simulink model "v13_na_ri_8r128dr_ver101"  (Simulink 7.6)
settings: Solver=ode45  StartTime=0.0  StopTime=10000  RelTol=1e-3
libraries: xbsIndex_r4 x1533, xps_library x40, casper_library_misc x20, dspsigops x16, simulink x7, casper_library_flow_control x4
(part 1 of 15)
settings Model: Creator=dave  LastModifiedBy=chenhong
settings OptimizationCC: InitFltsAndDblsToZero=on
settings DebuggingCC: StrictBusMsg=Warning

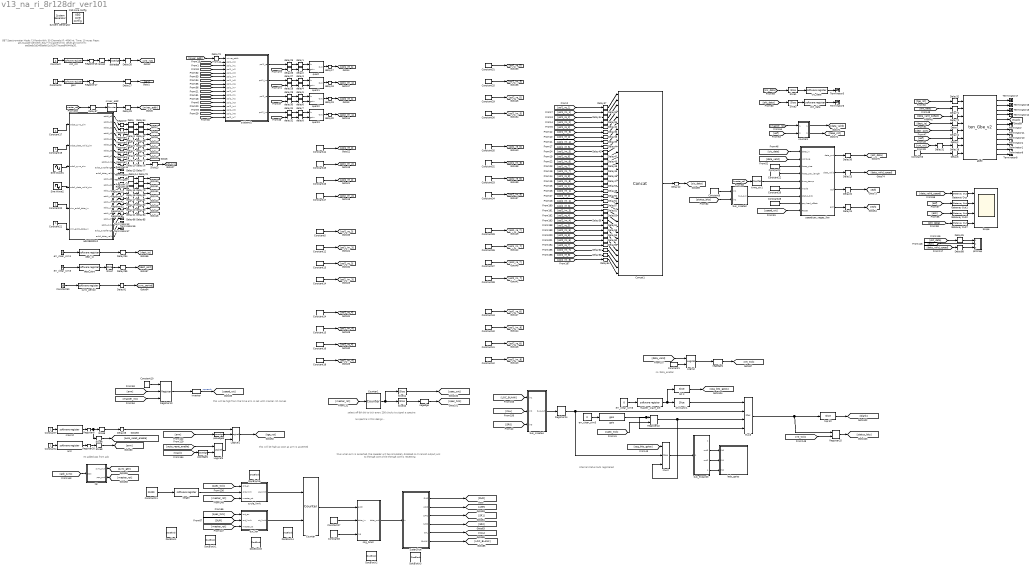
== system v13_na_ri_8r128dr_ver101 ==
  note: GBT Spectrometer
      | Mode: 13
      | Bandwidth: 30
      | Channels/IF: 4096
      | Int. Time: 10 msec
      | Repo: git://casper.berkeley.edu/~mwagner/mlib_devel.git
      | Commit: ae0be6d1d34f5befa01c913b77ecae840440e2f1
  note: internal status buts registered
  note: r6: added pps from adc
  note: this will be high from the time arm is set until master rst comes
  note: this will be high as soon as arm is asserted
  note: thus when arm is asserted, the speader will be completely disabled so it cannot output junk to the tge core while the tge core is resetting
  note: select off 8th bit to tick every 256 clocks to signal a spectra
  note: no 'data_enable'
  note: no spectra in this design...
  " System Generator" : xbsIndex_r4/ System Generator  Tag=genX  SystemSampleTime=-1  xilinxfamily=virtex5  part=xc5vsx95t  speed=-1  package=ff1136  synthesis_tool=XST  clock_wrapper=Clock Enables  directory=./mode13_1sub_no_cic/sysgen  proj_type=Project Navigator  Synth_file=XST Defaults  Impl_file=ISE Defaults  testbench=off  simulink_period=1  sysclk_period=6.6667  dcm_input_clock_period=100  incr_netlist=off  trim_vbits=Everywhere in SubSystem  dbl_ovrd=According to Block Masks  core_generation=According to Block Masks  run_coregen=off  deprecated_control=off  eval_field=0  block_type=sysgen
  Concat1 : xbsIndex_r4/Concat  num_inputs=32  dbl_ovrd=off  block_type=concat
  Constant{,3..4,7,17,19..21} (8 alike) : Constant
  Constant1 : xbsIndex_r4/Constant  const=1  gui_display_data_type=Boolean  arith_type=Boolean  n_bits=8  bin_pt=0  preci_type=Single  exp_width=8  frac_width=24  explicit_period=on  period=1  equ=P=C  opselect=C  inp2=PCIN>>17  opr=+  inp1=P  carry=CIN  dbl_ovrd=off  block_type=constant
  Constant10 : xbsIndex_r4/Constant  const=1  gui_display_data_type=Fixed-point  arith_type=Boolean  n_bits=16  bin_pt=0  preci_type=Single  exp_width=8  frac_width=24  explicit_period=on  period=1  equ=P=C  opselect=C  inp2=PCIN>>17  opr=+  inp1=P  carry=CIN  dbl_ovrd=off  block_type=constant
      settings: block_version=11.4
  Constant11 : Constant  Value=21200  VectorParams1D=off
  Constant{5,12..16,18,24..25,29..47} (28 alike) : xbsIndex_r4/Constant  const=0  gui_display_data_type=Fixed-point  arith_type=Unsigned  n_bits=8  bin_pt=0  preci_type=Single  exp_width=8  frac_width=24  explicit_period=on  period=1  equ=P=C  opselect=C  inp2=PCIN>>17  opr=+  inp1=P  carry=CIN  dbl_ovrd=off  block_type=constant
  Constant2 : xbsIndex_r4/Constant  const=8192+4*8  gui_display_data_type=Fixed-point  arith_type=Unsigned  n_bits=40  bin_pt=0  preci_type=Single  exp_width=8  frac_width=24  explicit_period=on  period=1  equ=P=C  opselect=C  inp2=PCIN>>17  opr=+  inp1=P  carry=CIN  dbl_ovrd=off  block_type=constant
  Constant22 : xbsIndex_r4/Constant  const=8192  gui_display_data_type=Fixed-point  arith_type=Unsigned  n_bits=40  bin_pt=0  preci_type=Single  exp_width=8  frac_width=24  explicit_period=on  period=1  equ=P=C  opselect=C  inp2=PCIN>>17  opr=+  inp1=P  carry=CIN  dbl_ovrd=off  block_type=constant
  Constant26 : xbsIndex_r4/Constant  const=13  gui_display_data_type=Fixed-point  arith_type=Unsigned  n_bits=40  bin_pt=0  preci_type=Single  exp_width=8  frac_width=24  explicit_period=on  period=1  equ=P=C  opselect=C  inp2=PCIN>>17  opr=+  inp1=P  carry=CIN  dbl_ovrd=off  block_type=constant
  Constant27 : xbsIndex_r4/Constant  const=0  gui_display_data_type=Fixed-point  arith_type=Unsigned  n_bits=32  bin_pt=0  preci_type=Single  exp_width=8  frac_width=24  explicit_period=on  period=1  equ=P=C  opselect=C  inp2=PCIN>>17  opr=+  inp1=P  carry=CIN  dbl_ovrd=off  block_type=constant
      settings: block_version=11.3
  Constant28 : xbsIndex_r4/Constant  const=0  gui_display_data_type=Fixed-point  arith_type=Unsigned  n_bits=40  bin_pt=0  preci_type=Single  exp_width=8  frac_width=24  explicit_period=on  period=1  equ=P=C  opselect=C  inp2=PCIN>>17  opr=+  inp1=P  carry=CIN  dbl_ovrd=off  block_type=constant
  Constant50 : Constant  Value=16
  Constant6 : xbsIndex_r4/Constant  const=0  gui_display_data_type=Fixed-point  arith_type=Boolean  n_bits=1  bin_pt=0  preci_type=Single  exp_width=8  frac_width=24  explicit_period=on  period=1  equ=P=C  opselect=C  inp2=PCIN>>17  opr=+  inp1=P  carry=CIN  dbl_ovrd=off  block_type=constant
  Constant8 : xbsIndex_r4/Constant  const=0  gui_display_data_type=Fixed-point  arith_type=Boolean  n_bits=32  bin_pt=0  preci_type=Single  exp_width=8  frac_width=24  explicit_period=on  period=1  equ=P=C  opselect=C  inp2=PCIN>>17  opr=+  inp1=P  carry=CIN  dbl_ovrd=off  block_type=constant
      settings: block_version=11.3
  Constant9 : xbsIndex_r4/Constant  const=0  gui_display_data_type=Fixed-point  arith_type=Unsigned  n_bits=36  bin_pt=0  preci_type=Single  exp_width=8  frac_width=24  explicit_period=on  period=1  equ=P=C  opselect=C  inp2=PCIN>>17  opr=+  inp1=P  carry=CIN  dbl_ovrd=off  block_type=constant
  Counter : xbsIndex_r4/Counter  cnt_type=Free Running  cnt_to=Inf  operation=Up  start_count=0  cnt_by_val=1  arith_type=Unsigned  n_bits=8  bin_pt=0  load_pin=off  rst=on  en=on  explicit_period=on  period=1  dbl_ovrd=off  use_behavioral_HDL=off  implementation=DSP48  block_type=counter
      settings: block_version=11.3
  Counter1 : xbsIndex_r4/Counter  cnt_type=Free Running  cnt_to=Inf  operation=Up  start_count=0  cnt_by_val=1  arith_type=Unsigned  n_bits=48  bin_pt=0  load_pin=off  rst=on  en=off  explicit_period=on  period=1  dbl_ovrd=off  use_behavioral_HDL=off  implementation=DSP48  block_type=counter
      settings: block_version=11.3
  Delay{,3..5,7..10,13..17,19..24,30..31,66} (22 alike) : xbsIndex_r4/Delay  rst=off  infoeditControl=Selection of Reset will increase slice count due to use of real FFs and instead of SRLs  en=off  latency=1  dbl_ovrd=off  reg_retiming=on  block_type=delay
      Delay settings: block_version=11.4
      Delay10 settings: block_version=11.4
      Delay13 settings: block_version=11.4
      Delay14 settings: block_version=11.4
      Delay15 settings: block_version=11.4
      Delay16 settings: block_version=11.4
      Delay17 settings: block_version=11.4
      Delay19 settings: block_version=11.4
      Delay20 settings: block_version=11.4
      Delay21 settings: block_version=11.4
      Delay3 settings: block_version=11.4
      Delay4 settings: block_version=11.4
      Delay5 settings: block_version=11.4
      Delay66 settings: block_version=11.4
      Delay7 settings: block_version=11.4
      Delay8 settings: block_version=11.4
      Delay9 settings: block_version=11.4
  Delay{1..2,6,11,18,28,32,71..72} (9 alike) : xbsIndex_r4/Delay  rst=off  infoeditControl=Selection of Reset will increase slice count due to use of real FFs and instead of SRLs  en=off  latency=8  dbl_ovrd=off  reg_retiming=on  block_type=delay
  Delay{12,33..53,55..65} (33 alike) : xbsIndex_r4/Delay  rst=off  infoeditControl=Selection of Reset will increase slice count due to use of real FFs and instead of SRLs  en=off  latency=4  dbl_ovrd=off  reg_retiming=on  block_type=delay
      settings: block_version=11.4
  Delay25 : xbsIndex_r4/Delay  rst=off  infoeditControl=Selection of Reset will increase slice count due to use of real FFs and instead of SRLs  en=off  latency=12  dbl_ovrd=off  reg_retiming=on  block_type=delay
  Delay{26..27,29,73} (4 alike) : xbsIndex_r4/Delay  rst=off  infoeditControl=Selection of Reset will increase slice count due to use of real FFs and instead of SRLs  en=off  latency=2  dbl_ovrd=off  reg_retiming=on  block_type=delay
      settings: block_version=11.4
  Delay{87,91,294..295} (4 alike) : xbsIndex_r4/Delay  rst=off  infoeditControl=Selection of Reset will increase slice count due to use of real FFs and instead of SRLs  en=off  latency=1  dbl_ovrd=off  reg_retiming=off  block_type=delay
      settings: block_version=11.4
  Delay{54,67..70,74..85} (17 alike) : xbsIndex_r4/Delay  rst=off  infoeditControl=Selection of Reset will increase slice count due to use of real FFs and instead of SRLs  en=off  latency=3  dbl_ovrd=off  reg_retiming=on  block_type=delay
      settings: block_version=11.4
  Delay88 : xbsIndex_r4/Delay  rst=off  infoeditControl=Selection of Reset will increase slice count due to use of real FFs and instead of SRLs  en=off  latency=12  dbl_ovrd=off  reg_retiming=on  block_type=delay
  DocBlock{,1..4,6,8} (7 alike) : simulink/Model-Wide Utilities/DocBlock  SystemSampleTime=-1  DocumentType=Text
  Gateway Out{,1..2,7} (4 alike) : xbsIndex_r4/Gateway Out  hdl_port=on  timing_constraint=None  locs_specified=off  LOCs={}  block_type=gatewayout
      settings: infoedit="Gateway out block.  Converts Xilinx fixed point inputs into ouputs of type Simulink integer, double, or fixed point.<br><br>Hardware notes:  In hardware these blocks become top level output ports or are discarded, depending on how they are configured."  block_version=11.4
  Inverter : xbsIndex_r4/Inverter  en=off  latency=0  dbl_ovrd=off  block_type=inv
      settings: block_version=11.4
  Logical : xbsIndex_r4/Logical  logical_function=AND  inputs=2  en=off  latency=0  precision=Full  arith_type=Unsigned  n_bits=16  bin_pt=0  align_bp=on  dbl_ovrd=off  block_type=logical
      settings: block_version=11.4
  Logical2 : xbsIndex_r4/Logical  logical_function=OR  inputs=3  en=off  latency=0  precision=Full  arith_type=Unsigned  n_bits=8  bin_pt=2  align_bp=on  dbl_ovrd=off  block_type=logical
      settings: xl_area=[0, 0, 0, 0, 0, 0, 0]
  Logical3 : xbsIndex_r4/Logical  logical_function=AND  inputs=2  en=off  latency=1  precision=Full  arith_type=Unsigned  n_bits=8  bin_pt=2  align_bp=on  dbl_ovrd=off  block_type=logical
      settings: xl_area=[0, 0, 0, 0, 0, 0, 0]
  Mux2 : xbsIndex_r4/Mux  inputs=2  en=off  latency=0  precision=Full  arith_type=Unsigned  n_bits=16  bin_pt=14  quantization=Truncate  overflow=Wrap  dbl_ovrd=off  block_type=mux
  Mux3 : xbsIndex_r4/Mux  inputs=3  en=off  latency=0  precision=Full  arith_type=Unsigned  n_bits=16  bin_pt=14  quantization=Truncate  overflow=Wrap  dbl_ovrd=off  block_type=mux
  Register{,1..8,12..13,16..17,20,24,42..44,46..47,52,61} (22 alike) : xbsIndex_r4/Register  init=0  rst=off  en=off  dbl_ovrd=off  block_type=register
      settings: block_version=11.4
  Register10 : xbsIndex_r4/Register  init=0  rst=on  en=on  dbl_ovrd=off  block_type=register
      settings: block_version=11.4
  Register22 : xbsIndex_r4/Register  init=0  rst=off  en=on  dbl_ovrd=off  block_type=register
      settings: block_version=11.4
  Register25 : xbsIndex_r4/Register  init=0  rst=off  en=on  dbl_ovrd=off  block_type=register
      settings: block_version=11.4
  Scope : Scope  NumInputPorts=4
      settings: TimeRange=6000  YMin=0~-1~0~0  YMax=1~1~300000000000000~1e+19  MaxDataPoints=1000000
  Sine Wave1 : Sin  Amplitude=.9  Frequency=2.*pi/4.  SampleTime=.0625
  Sine Wave2 : Sin  Amplitude=.9  Frequency=pi/2  Phase=2*pi*240/200  SampleTime=.0625
  Slice1 : xbsIndex_r4/Slice  nbits=32  boolean_output=off  mode=Lower Bit Location + Width  bit1=0  base1=MSB of Input  bit0=0  base0=LSB of Input  dbl_ovrd=off  block_type=slice
  Slice10 : xbsIndex_r4/Slice  nbits=1  boolean_output=on  mode=Lower Bit Location + Width  bit1=7  base1=MSB of Input  bit0=7  base0=LSB of Input  dbl_ovrd=off  block_type=slice
      settings: block_version=10.1.3
  Slice11 : xbsIndex_r4/Slice  nbits=40  boolean_output=off  mode=Lower Bit Location + Width  bit1=0  base1=MSB of Input  bit0=8  base0=LSB of Input  dbl_ovrd=off  block_type=slice
      settings: block_version=10.1.3
  Slice{2,6,8,13} (4 alike) : xbsIndex_r4/Slice  nbits=1  boolean_output=on  mode=Lower Bit Location + Width  bit1=0  base1=MSB of Input  bit0=0  base0=LSB of Input  dbl_ovrd=off  block_type=slice
      Slice13 settings: infoedit="Extracts a given range of bits from each input sample and presents it at the output.  The output type is ordinarily unsigned with binary point at zero, but can be Boolean when the slice is one bit wide.<P><P>Hardware notes: In hardware this block costs nothing."  block_version=10.1.3
      Slice2 settings: block_version=10.1.3
      Slice6 settings: block_version=10.1.3
      Slice8 settings: block_version=10.1.3
  Slice3 : xbsIndex_r4/Slice  nbits=32  boolean_output=off  mode=Lower Bit Location + Width  bit1=0  base1=MSB of Input  bit0=0  base0=LSB of Input  dbl_ovrd=off  block_type=slice
  Slice7 : xbsIndex_r4/Slice  nbits=16  boolean_output=off  mode=Lower Bit Location + Width  bit1=0  base1=MSB of Input  bit0=0  base0=LSB of Input  dbl_ovrd=off  block_type=slice
      settings: infoedit="Extracts a given range of bits from each input sample and presents it at the output.  The output type is ordinarily unsigned with binary point at zero, but can be Boolean when the slice is one bit wide.<P><P>Hardware notes: In hardware this block costs nothing."  block_version=10.1.3
  Slice9 : xbsIndex_r4/Slice  nbits=1  boolean_output=on  mode=Lower Bit Location + Width  bit1=0  base1=MSB of Input  bit0=1  base0=LSB of Input  dbl_ovrd=off  block_type=slice
      settings: block_version=10.1.3
  SuperSlice : Subsystem  AncestorBlock=gavrt_library/SuperSlice  mask(superslice){slices=[27,1,1,1,1,1]}
      mask prompts: slices "Slice pattern"
      mask help:
      | Slices the input word into consecutive outputs of arbitrary width.
      | Slice pattern should be a vector with each entry representing the width of that particular slice. The first entry is the MSB slice and the last is the LSB slice.
      | If a slice is given width -1, that slice will have a boolean output.
      | Ex: -1*ones(1,32)  -- Slice a 32 bit word into seperate boolean flags
      | [16, 8, -1*ones(1,8)] -- Slice a 32 bit word into the MSW, a byte, and the least significant 8 bits
      mask init:
      | superslice_init(gcb, 'slices', slices);
  Terminator{,1,3..13,16..17,43,46..47} (18 alike) : Terminator
  XSG core config : xps_library/XSG core config  Tag=xps:xsg  SystemSampleTime=-1  hw_sys=ROACH:sx95t  ibob_linux=off  clk_src=adc0_clk  iBOB_clk_src=sys_clk  BEE2_ctrl_clk_src=sys_clk  BEE2_usr_clk_src=sys_clk  ROACH_clk_src=adc0_clk  ROACH2_clk_src=adc0_clk  CORR_clk_src=sys_clk  gpio_clk_io_group=iBOB:sma  gpio_clk_bit_index=0  clk_rate=150  sample_period=1  synthesis_tool=XST  mpc_type=powerpc440_ext
  adc083000x2 : Subsystem  Tag=xps:adc083000x2  AncestorBlock=xps_library/adc083000x2  mask(adc_083000x2){use_adc0=on, use_adc1=on, demux_adc=off, clock_sync=off, verbose=off, using_ctrl=on, adc_clk_rate=1000, sample_period=1, bit_width=8}
      mask prompts: use_adc0 "Use ZD0K0?"; use_adc1 "Use ZDOK1?"; demux_adc "Demux ADCs by 2?"; clock_sync "Interleave 2 ADC boards?"; verbose "Verbose Block Creation?"; using_ctrl "Add ports for control registers?"; adc_clk_rate "ADC clock rate (MHz)"; sample_period "Sample Period"; bit_width "Output Bit Width"
      mask help:
      | The ADC block converts analog inputs to digital outputs.
      | Every clock cycle, the inputs are sampled and digitized to
      | 8 bit binary point numbers in the range of [-1, 1).
      |
      | Online block-specific documentation: http://casper.berkeley.edu/wiki/Adc083000
      mask init:
      | adc083000_mask;
      mask Help: eval('xlWeb([getenv(''MLIB_ROOT''), ''\doc\html\sys_adc\sys_adc.html''])')
  arm : xps_library/software register  Tag=xps:sw_reg  SystemSampleTime=-1  io_dir=From Processor  arith_type=Unsigned  bitwidth=32  bin_pt=0  sample_period=1  gw_name=v13_na_ri_8r128dr_ver101_arm_user_data_out
      link override convert: arith_type=Unsigned  n_bits=32  bin_pt=0
      link override r_spec_2048_r102_cnt_rst_user_data_out: arith_type=Unsigned  n_bits=32  bin_pt=0  period=1
      link override r_spec_2048_r102_nosim_cnt_rst_user_data_out: arith_type=Unsigned  n_bits=32  bin_pt=0  period=1
      link override r_spec_2048_r103_cnt_rst_user_data_out: arith_type=Unsigned  n_bits=32  bin_pt=0  period=1
      link override roach_spec_no10gbe_sync_cnt_rst_user_data_out: arith_type=Unsigned  n_bits=32  bin_pt=0  period=1
      link override roach_spec_rst_cnt_user_data_out: arith_type=Unsigned  n_bits=32  bin_pt=0  period=1
      link override roach_spec_rst_sync_cnt_user_data_out: arith_type=Unsigned  n_bits=32  bin_pt=0  period=1
  bit 0 and 1 : xbsIndex_r4/Slice  nbits=2  boolean_output=off  mode=Lower Bit Location + Width  bit1=0  base1=MSB of Input  bit0=0  base0=LSB of Input  dbl_ovrd=off  block_type=slice
  bit 2 : xbsIndex_r4/Slice  nbits=1  boolean_output=on  mode=Lower Bit Location + Width  bit1=0  base1=MSB of Input  bit0=2  base0=LSB of Input  dbl_ovrd=off  block_type=slice
  bus_create2 : Subsystem  AncestorBlock=casper_library_flow_control/bus_create  mask(bus_create){inputNum=2}
      mask prompts: inputNum "Number of inputs:"
      mask init:
      | bus_create_init(gcb,...
      |     'inputNum', inputNum);
  bus_create4 : Subsystem  AncestorBlock=casper_library_flow_control/bus_create  mask(bus_create){inputNum=3}
      mask prompts: inputNum "Number of inputs:"
      mask init:
      | bus_create_init(gcb,...
      |     'inputNum', inputNum);
  bus_expand3 : Subsystem  AncestorBlock=casper_library_flow_control/bus_expand  mask(bus_expand){mode=divisions of equal size, outputNum=4, outputWidth=1, outputBinaryPt=0, outputArithmeticType=2, outputToWorkspace=off, variablePrefix=out, outputToModelAsWell=on}
      mask prompts: outputNum "Number of outputs:"; outputWidth "Output width:"; outputBinaryPt "Output binary point position:"; outputArithmeticType "Output arithmetic type: (Unsigned=0, Signed=1, Boolean=2)"; outputToWorkspace "Output to workspace?"; variablePrefix "Variable name prefix:"; outputToModelAsWell "Output to model as well?"
      mask help:
      | Expands a 'bus' made using a bus_create or Xilinx
      | concat block.
      | The input will be divided into the specified number
      | of outputs with the specified arithmetic types.
      | Divisions are made from most significant bit.
      mask init:
      | bus_expand_init(gcb,...
      |     'mode', mode, ...
      |     'outputNum', outputNum, ...
      |     'outputWidth', outputWidth, ...
      |     'outputBinaryPt', outputBinaryPt, ...
      |     'outputArithmeticType', outputArithmeticType, ...
      |     'outputToWorkspace', outputToWorkspace, ...
      |     'variablePrefix', variablePrefix, ...
      |     'outputToModelAsWell', outputToModelAsWell);
      settings: AttributesFormatString=4 outputs
      mask StyleString: popup(divisions of equal size|divisions of arbitrary size),edit,edit,edit,edit,checkbox,edit,checkbox
      mask CallbackString: bus_expand_callback();||bus_expand_callback();|||bus_expand_callback();||
  cic_data : xps_library/software register  Tag=xps:sw_reg  SystemSampleTime=-1  io_dir=To Processor  arith_type=Unsigned  bitwidth=32  bin_pt=0  sample_period=1  gw_name=v13_na_ri_8r128dr_ver101_cic_data_user_data_in
  cnt_rst : xps_library/software register  Tag=xps:sw_reg  SystemSampleTime=-1  io_dir=From Processor  arith_type=Unsigned  bitwidth=32  bin_pt=0  sample_period=1  gw_name=v13_na_ri_8r128dr_ver101_cnt_rst_user_data_out
  counters : Subsystem
  cycle_limit : Subsystem
  delay{1..8} (8 alike) : xbsIndex_r4/Delay  rst=off  infoeditControl=Selection of Reset will increase slice count due to use of real FFs and instead of SRLs  en=off  latency=4  dbl_ovrd=off  reg_retiming=on  block_type=delay
      settings: block_version=11.4
  delay{17..20} (4 alike) : xbsIndex_r4/Delay  rst=off  infoeditControl=Selection of Reset will increase slice count due to use of real FFs and instead of SRLs  en=off  latency=3  dbl_ovrd=off  reg_retiming=on  block_type=delay
      settings: block_version=11.4
  delay{25..32} (8 alike) : xbsIndex_r4/Delay  rst=off  infoeditControl=Selection of Reset will increase slice count due to use of real FFs and instead of SRLs  en=off  latency=1  dbl_ovrd=off  reg_retiming=on  block_type=delay
      settings: block_version=11.4
  dest_ip : xps_library/software register  Tag=xps:sw_reg  SystemSampleTime=-1  io_dir=From Processor  arith_type=Unsigned  bitwidth=32  bin_pt=0  sample_period=1  gw_name=v13_na_ri_8r128dr_ver101_dest_ip_user_data_out
  dest_port : xps_library/software register  Tag=xps:sw_reg  SystemSampleTime=-1  io_dir=From Processor  arith_type=Unsigned  bitwidth=32  bin_pt=0  sample_period=1  gw_name=v13_na_ri_8r128dr_ver101_dest_port_user_data_out
  dur_len : Subsystem
  err_clear_sim{1,3,5..6} (4 alike) : Constant  Value=0
  gain : xps_library/software register  Tag=xps:sw_reg  SystemSampleTime=-1  io_dir=From Processor  arith_type=Unsigned  bitwidth=32  bin_pt=0  sample_period=1  gw_name=v13_na_ri_8r128dr_ver101_gain_user_data_out
  gbe0 : xps_library/ten_Gbe_v2  Tag=xps:tengbe_v2  SystemSampleTime=-1  port=0  rx_dist_ram=on  large_frames=on  show_param=on  pre_emph=3  swing=800  fab_en=off  fab_mac=0  fab_ip=0  fab_udp=0  fab_gate=0  cpu_rx_en=on  cpu_tx_en=on
  gpio : xps_library/gpio  Tag=xps:gpio  SystemSampleTime=-1  io_group=ROACH:gpiob  io_dir=in  arith_type=Unsigned  bitwidth=4  bin_pt=0  bit_index=[1:4]  sample_period=1  use_single_ended=off  use_ddr=off  reg_iob=on  reg_clk_phase=0  termination=None
      link override ssg_speader_r2_gpio_gateway: arith_type=Unsigned  n_bits=4  bin_pt=0  period=sample_period
  leds_gpios : Subsystem
  length : xps_library/software register  Tag=xps:sw_reg  SystemSampleTime=-1  io_dir=From Processor  arith_type=Unsigned  bitwidth=32  bin_pt=0  sample_period=1  gw_name=v13_na_ri_8r128dr_ver101_length_user_data_out
      link override ssg_speader_r2_length_user_data_out: arith_type=Unsigned  n_bits=32  bin_pt=0  period=1
  manrst : xps_library/software register  Tag=xps:sw_reg  SystemSampleTime=-1  io_dir=From Processor  arith_type=Unsigned  bitwidth=32  bin_pt=0  sample_period=1  gw_name=v13_na_ri_8r128dr_ver101_manrst_user_data_out
      link override convert: arith_type=Unsigned  n_bits=32  bin_pt=0
      link override r_spec_2048_r102_cnt_rst_user_data_out: arith_type=Unsigned  n_bits=32  bin_pt=0  period=1
      link override r_spec_2048_r102_nosim_cnt_rst_user_data_out: arith_type=Unsigned  n_bits=32  bin_pt=0  period=1
      link override r_spec_2048_r103_cnt_rst_user_data_out: arith_type=Unsigned  n_bits=32  bin_pt=0  period=1
      link override roach_spec_no10gbe_sync_cnt_rst_user_data_out: arith_type=Unsigned  n_bits=32  bin_pt=0  period=1
      link override roach_spec_rst_cnt_user_data_out: arith_type=Unsigned  n_bits=32  bin_pt=0  period=1
      link override roach_spec_rst_sync_cnt_user_data_out: arith_type=Unsigned  n_bits=32  bin_pt=0  period=1
  master_slave_sel : xps_library/software register  Tag=xps:sw_reg  SystemSampleTime=-1  io_dir=From Processor  arith_type=Unsigned  bitwidth=32  bin_pt=0  sample_period=1  gw_name=v13_na_ri_8r128dr_ver101_master_slave_sel_user_data_out
      link override ssg_speader_r2_master_slave_sel_user_data_out: arith_type=Unsigned  n_bits=32  bin_pt=0  period=1
  mixer_addr : xbsIndex_r4/Counter  cnt_type=Free Running  cnt_to=Inf  operation=Up  start_count=0  cnt_by_val=1  arith_type=Unsigned  n_bits=9  bin_pt=0  load_pin=off  rst=on  en=off  explicit_period=on  period=1  dbl_ovrd=off  use_behavioral_HDL=off  implementation=Fabric  block_type=counter
      settings: block_version=11.4
  negedge : casper_library_misc/negedge  Tag=Rising Edge Detector  SystemSampleTime=-1
  negedge1 : casper_library_misc/negedge  Tag=Rising Edge Detector  SystemSampleTime=-1
  pkt_data : xps_library/software register  Tag=xps:sw_reg  SystemSampleTime=-1  io_dir=To Processor  arith_type=Unsigned  bitwidth=32  bin_pt=0  sample_period=1  gw_name=v13_na_ri_8r128dr_ver101_pkt_data_user_data_in
  pktsnap : Subsystem  AncestorBlock=casper_library_scopes/snap64  mask(snap64){nsamples=11}
      mask prompts: nsamples "No. of samples(2^?)"
      mask help:
      | The <i>snap</i> block provides a packaged solution to capturing data from
      | the FPGA fabric and making it accessible from the CPU.  The block comes in
      | 2 flavors: <i>snap</i> (which captures to a 32 bit wide Shared BRAM), and
      | <i>snap64</i> (which captures to 2x32 bit wide Shared BRAMs to effect a
      | 64 bit capture).
      mask Help: eval('xlWeb(''http://casper.berkeley.edu/wiki/Snap'')')
  posedge : casper_library_misc/posedge  Tag=Rising Edge Detector  SystemSampleTime=-1
  posedge1 : casper_library_misc/posedge  Tag=Rising Edge Detector  SystemSampleTime=-1
  posedge3 : casper_library_misc/posedge  Tag=Rising Edge Detector  SystemSampleTime=-1
  quant : Subsystem  mask{n_bits=45, bin_pt=22, mult_latency=10}
      mask prompts: n_bits "Bit Width"; bin_pt "Binary Point"; mult_latency "Multiplier Latency"
  quant1 : Subsystem  mask{n_bits=45, bin_pt=22, mult_latency=10}
      mask prompts: n_bits "Bit Width"; bin_pt "Binary Point"; mult_latency "Multiplier Latency"
  quant2 : Subsystem  mask{n_bits=45, bin_pt=22, mult_latency=10}
      mask prompts: n_bits "Bit Width"; bin_pt "Binary Point"; mult_latency "Multiplier Latency"
  quant3 : Subsystem  mask{n_bits=45, bin_pt=22, mult_latency=10}
      mask prompts: n_bits "Bit Width"; bin_pt "Binary Point"; mult_latency "Multiplier Latency"
  sg : Subsystem
  speadizer_vegas_lbw : Subsystem  AncestorBlock=gbt_library/speadizer_vegas_lbw  mask(speadizer_vegas){spead_msw=24, spead_lsw=40, num_items=4, num_payloads=1, pktzr_waste_time=3, data_input_width=256}
      mask prompts: spead_msw "Num bits for SPEAD Item ID"; spead_lsw "Num bits for SPEAD Item value"; num_items "Number of items (+5)"; num_payloads "Number of packets per heap"; pktzr_waste_time "Packetizer waste time"; data_input_width "Data input width"
      mask help:
      | The size of the heap and payload are in Bytes.
      | If there is one packet per heap then payload_offset is 0.
      | All the input must be not longer than 40 bits.
      mask init:
      | speadizer_vegas_init(gcb, 'spead_msw', spead_msw, 'spead_lsw', spead_lsw, 'num_items', num_items, 'num_payloads', num_payloads, 'pktzr_waste_time', pktzr_waste_time, 'data_input_width', data_input_width)
  ssg_bram : Subsystem  Tag=xps:bram  AncestorBlock=xps_library/Shared BRAM  mask{arith_type=Unsigned, addr_width=11, data_width=32, data_bin_pt=0, init_vals=[12801,121600,3201,3203,57602,3203,3201,121600,12801,12805,121604,3205,3207,57606,3207,3205,121604,12805,0:0], sample_rate=1}
      mask prompts: arith_type "Output Data Type"; addr_width "Address width"; data_width "Data Width"; data_bin_pt "Data Binary Point"; init_vals "Initial 32-bit values (simulation only)"; sample_rate "Sample rate"
      mask init:
      | shared_bram_mask;
      mask StyleString: popup(Unsigned|Signed  (2's comp)),edit,popup(8|16|32|64|128),edit,edit,edit
  subband1 : Subsystem  mask{mult_latency=3, add_latency=1}
      mask prompts: mult_latency "Multiplier Latency"; add_latency "Adder Latency"
  sync_period : xps_library/software register  Tag=xps:sw_reg  SystemSampleTime=-1  io_dir=From Processor  arith_type=Unsigned  bitwidth=32  bin_pt=0  sample_period=1  gw_name=v13_na_ri_8r128dr_ver101_sync_period_user_data_out
  time_ctr1 : xbsIndex_r4/Counter  cnt_type=Free Running  cnt_to=Inf  operation=Up  start_count=0  cnt_by_val=1  arith_type=Unsigned  n_bits=40  bin_pt=0  load_pin=off  rst=on  en=off  explicit_period=on  period=1  dbl_ovrd=off  use_behavioral_HDL=off  implementation=Fabric  block_type=counter
      settings: block_version=11.4
  top bit : xbsIndex_r4/Slice  nbits=1  boolean_output=on  mode=Upper Bit Location + Width  bit1=0  base1=MSB of Input  bit0=0  base0=LSB of Input  dbl_ovrd=off  block_type=slice
  wires:
  cnt_rst/1 -> Register16/1
  Constant/1 -> cnt_rst/1
  gain/1 -> Register17/1
  Register16/1 -> Slice2/1
  Slice2/1 -> posedge/1
  Register17/1 -> Delay27/1
  Register/1 -> Delay/1
  Sine Wave1/1 -> adc083000x2/3(adc1_sync_sim)
  Delay13/1 -> Delay77/1
  Register44/1 -> Delay13/1
  adc083000x2/9(adc0_sync) -> Register44/1
  adc083000x2/20(adc1_sync) -> Terminator43/1
  adc083000x2/21(adc1_outofrange) -> Terminator46/1
  adc083000x2/22(adc1_data_valid) -> Terminator47/1
  posedge/1 -> Delay26/1
  Sine Wave2/1 -> adc083000x2/4(adc1_data_valid_sim)
  Constant20/1 -> adc083000x2/5(sim_adc0_data_in)
  Constant21/1 -> adc083000x2/6(sim_adc1_data_in)
  adc083000x2/1(adc0_s0) -> Register/1
  adc083000x2/3(adc0_s2) -> Register1/1
  adc083000x2/5(adc0_s4) -> Register2/1
  adc083000x2/7(adc0_s6) -> Register3/1
  adc083000x2/8(adc0_s7) -> Register7/1
  adc083000x2/6(adc0_s5) -> Register6/1
  adc083000x2/2(adc0_s1) -> Register4/1
  adc083000x2/4(adc0_s3) -> Register5/1
  adc083000x2/10(adc0_outofrange) -> Terminator3/1
  Register12/1 -> Delay14/1
  Register46/1 -> Delay15/1
  Register13/1 -> Delay16/1
  Register47/1 -> Delay17/1
  Register42/1 -> Delay19/1
  Register52/1 -> Delay20/1
  Register43/1 -> Delay21/1
  Register61/1 -> Delay66/1
  mixer_addr/1 -> Delay29/1
  Delay/1 -> Delay54/1
  adc083000x2/11(adc0_data_valid) -> Terminator7/1
  adc083000x2/12(adc1_s0) -> Register12/1
  adc083000x2/13(adc1_s1) -> Register46/1
  adc083000x2/14(adc1_s2) -> Register13/1
  adc083000x2/15(adc1_s3) -> Register47/1
  adc083000x2/16(adc1_s4) -> Register42/1
  adc083000x2/17(adc1_s5) -> Register52/1
  adc083000x2/18(adc1_s6) -> Register43/1
  adc083000x2/19(adc1_s7) -> Register61/1
  [master_rst] -> Delay87/1
  Delay26/1 -> [cnt_rst]
  Delay27/1 -> [gain]
  Delay29/1 -> [mixer_addr]
  Delay87/1 -> mixer_addr/1
  Delay3/1 -> Delay67/1
  Register4/1 -> Delay3/1
  Delay4/1 -> Delay68/1
  Delay5/1 -> Delay69/1
  Register1/1 -> Delay4/1
  Register5/1 -> Delay5/1
  Register2/1 -> Delay7/1
  Delay7/1 -> Delay70/1
  Delay8/1 -> Delay74/1
  Register6/1 -> Delay8/1
  Delay9/1 -> Delay75/1
  Delay10/1 -> Delay76/1
  Register3/1 -> Delay9/1
  Register7/1 -> Delay10/1
  Delay14/1 -> Delay78/1
  Delay15/1 -> Delay79/1
  Delay16/1 -> Delay80/1
  Delay17/1 -> Delay81/1
  Delay19/1 -> Delay82/1
  Delay20/1 -> Delay83/1
  Delay21/1 -> Delay84/1
  Delay66/1 -> Delay85/1
  [mixer_addr] -> Delay73/1
  [a0] -> subband1/2(pol1_in0)
  [a1] -> subband1/3(pol1_in1)
  [a2] -> subband1/4(pol1_in2)
  [a3] -> subband1/5(pol1_in3)
  [a4] -> subband1/6(pol1_in4)
  [a5] -> subband1/7(pol1_in5)
  [a6] -> subband1/8(pol1_in6)
  [a7] -> subband1/9(pol1_in7)
  [b0] -> subband1/10(pol2_in0)
  [b1] -> subband1/11(pol2_in1)
  [b2] -> subband1/12(pol2_in2)
  [b3] -> subband1/13(pol2_in3)
  [b4] -> subband1/14(pol2_in4)
  [b5] -> subband1/15(pol2_in5)
  [b6] -> subband1/16(pol2_in6)
  [b7] -> subband1/17(pol2_in7)
  subband1/1(pol1_re) -> delay25/1
  [gain] -> delay28/1
  subband1/2(pol1_im) -> delay27/1
  quant/1(Out) -> delay17/1
  quant1/1(Out) -> delay18/1
  [gain] -> delay30/1
  subband1/3(pol2_re) -> delay29/1
  [gain] -> delay32/1
  subband1/4(pol2_im) -> delay31/1
  quant2/1(Out) -> delay19/1
  quant3/1(Out) -> delay20/1
  delay1/1 -> quant/1(In)
  delay3/1 -> quant1/1(In)
  delay5/1 -> quant2/1(In)
  delay7/1 -> quant3/1(In)
  Constant5/1 -> [pol1_re_3]
  Constant12/1 -> [pol1_im_3]
  Constant13/1 -> [pol2_re_3]
  Constant14/1 -> [pol2_im_3]
  Constant24/1 -> [pol1_re_4]
  Constant15/1 -> [pol1_im_4]
  Constant16/1 -> [pol2_re_4]
  Constant18/1 -> [pol2_im_4]
  Constant31/1 -> [pol1_re_5]
  Constant25/1 -> [pol1_im_5]
  Constant29/1 -> [pol2_re_5]
  Constant30/1 -> [pol2_im_5]
  Constant35/1 -> [pol1_re_6]
  Constant32/1 -> [pol1_im_6]
  Constant33/1 -> [pol2_re_6]
  Constant34/1 -> [pol2_im_6]
  Constant39/1 -> [pol1_re_7]
  Constant36/1 -> [pol1_im_7]
  Constant37/1 -> [pol2_re_7]
  Constant38/1 -> [pol2_im_7]
  Constant43/1 -> [pol1_re_8]
  Constant40/1 -> [pol1_im_8]
  Constant41/1 -> [pol2_re_8]
  Constant42/1 -> [pol2_im_8]
  delay4/1 -> quant1/2(gain)
  delay6/1 -> quant2/2(gain)
  delay8/1 -> quant3/2(gain)
  Delay37/1 -> Concat1/1
  Delay33/1 -> Concat1/2
  Delay34/1 -> Concat1/3
  Delay35/1 -> Concat1/4
  Delay40/1 -> Concat1/5
  Delay36/1 -> Concat1/6
  Delay38/1 -> Concat1/7
  Delay39/1 -> Concat1/8
  Delay44/1 -> Concat1/9
  Delay41/1 -> Concat1/10
  Delay42/1 -> Concat1/11
  Delay43/1 -> Concat1/12
  Delay48/1 -> Concat1/13
  Delay45/1 -> Concat1/14
  Delay46/1 -> Concat1/15
  Delay47/1 -> Concat1/16
  Delay53/1 -> Concat1/17
  Delay49/1 -> Concat1/18
  Delay50/1 -> Concat1/19
  Delay51/1 -> Concat1/20
  Delay57/1 -> Concat1/21
  Delay52/1 -> Concat1/22
  Delay55/1 -> Concat1/23
  Delay56/1 -> Concat1/24
  Delay61/1 -> Concat1/25
  Delay58/1 -> Concat1/26
  Delay59/1 -> Concat1/27
  Delay60/1 -> Concat1/28
  Delay65/1 -> Concat1/29
  Delay62/1 -> Concat1/30
  Delay63/1 -> Concat1/31
  Delay64/1 -> Concat1/32
  Delay73/1 -> subband1/1(mixer_addr)
  delay2/1 -> quant/2(gain)
  Concat1/1 -> Delay12/1
  Delay12/1 -> [cic_data]
  [pol1_re_1] -> Delay37/1
  [pol1_im_1] -> Delay33/1
  [pol1_re_2] -> Delay34/1
  [pol1_im_2] -> Delay35/1
  [pol1_re_3] -> Delay40/1
  [pol1_im_3] -> Delay36/1
  [pol1_re_4] -> Delay38/1
  [pol1_im_4] -> Delay39/1
  [pol1_re_5] -> Delay44/1
  [pol1_im_5] -> Delay41/1
  [pol1_re_6] -> Delay42/1
  [pol1_im_6] -> Delay43/1
  [pol1_re_7] -> Delay48/1
  [pol1_im_7] -> Delay45/1
  [pol1_re_8] -> Delay46/1
  [pol1_im_8] -> Delay47/1
  [pol2_re_1] -> Delay53/1
  [pol2_im_1] -> Delay49/1
  [pol2_re_2] -> Delay50/1
  [pol2_im_2] -> Delay51/1
  [pol2_re_3] -> Delay57/1
  [pol2_im_3] -> Delay52/1
  [pol2_re_4] -> Delay55/1
  [pol2_im_4] -> Delay56/1
  [pol2_re_5] -> Delay61/1
  [pol2_im_5] -> Delay58/1
  [pol2_re_6] -> Delay59/1
  [pol2_im_6] -> Delay60/1
  [pol2_re_7] -> Delay65/1
  [pol2_im_7] -> Delay62/1
  [pol2_re_8] -> Delay63/1
  [pol2_im_8] -> Delay64/1
  delay28/1 -> delay4/1
  delay30/1 -> delay6/1
  delay32/1 -> delay8/1
  delay26/1 -> delay2/1
  [gain] -> delay26/1
  delay25/1 -> delay1/1
  delay27/1 -> delay3/1
  delay29/1 -> delay5/1
  delay31/1 -> delay7/1
  Constant44/1 -> [pol1_re_2]
  Constant45/1 -> [pol1_im_2]
  Constant46/1 -> [pol2_re_2]
  Constant47/1 -> [pol2_im_2]
  Delay54/1 -> [a0]
  Delay67/1 -> [a1]
  Delay68/1 -> [a2]
  Delay69/1 -> [a3]
  Delay70/1 -> [a4]
  Delay74/1 -> [a5]
  Delay75/1 -> [a6]
  Delay76/1 -> [a7]
  Delay77/1 -> Slice13/1
  Delay78/1 -> [b0]
  Delay79/1 -> [b1]
  Delay80/1 -> [b2]
  Delay81/1 -> [b3]
  Delay82/1 -> [b4]
  Delay83/1 -> [b5]
  Delay84/1 -> [b6]
  Delay85/1 -> [b7]
  Constant3/1 -> gain/1
  Constant17/1 -> adc083000x2/1(adc0_sync_sim)
  Constant19/1 -> adc083000x2/2(adc0_data_valid_sim)
  dest_port/1 -> Slice7/1
  err_clear_sim3/1 -> dest_port/1
  Slice7/1 -> Delay295/1
  Delay294/1 -> [dest_ip]
  Delay295/1 -> [dest_port]
  err_clear_sim1/1 -> dest_ip/1
  dest_ip/1 -> Delay294/1
  Constant50/1 -> sync_period/1
  sync_period/1 -> Delay91/1
  Constant6/1 -> Delay6/1
  [tge_rst] -> Delay32/1
  [eof] -> counters/2(In2)
  [master_rst] -> counters/1(In1)
  counters/2(Out2) -> [heap_cnt]
  Gateway Out/1 -> Scope/1
  Gateway Out2/1 -> Scope/3
  Gateway Out1/1 -> Scope/2
  [eoh] -> Gateway Out2/1
  [data_valid_spead] -> Gateway Out/1
  [eof] -> Gateway Out1/1
  counters/1(Out1) -> [data_valid]
  speadizer_vegas_lbw/1(data_out) -> Delay30/1
  speadizer_vegas_lbw/2(data_valid) -> Delay23/1
  [cic_data] -> speadizer_vegas_lbw/1(data_in)
  Constant2/1 -> speadizer_vegas_lbw/3(heap_size)
  Constant22/1 -> speadizer_vegas_lbw/4(heap_pay_length)
  time_ctr1/1 -> speadizer_vegas_lbw/5(time_stamp)
  Constant28/1 -> speadizer_vegas_lbw/8(payload_offset)
  Constant26/1 -> speadizer_vegas_lbw/6(mode)
  [spead_rst] -> speadizer_vegas_lbw/9(Reset)
  [data_valid] -> speadizer_vegas_lbw/2(valid_re)
  Gateway Out7/1 -> Scope/4
  [pkt_data] -> Gateway Out7/1
  [pkt_data] -> Delay71/1
  [dest_ip] -> Delay1/1
  [dest_port] -> Delay11/1
  cic_data/1 -> Terminator5/1
  pkt_data/1 -> Terminator6/1
  Slice1/1 -> cic_data/1
  [cic_data] -> Slice1/1
  Slice3/1 -> pkt_data/1
  [pkt_data] -> Slice3/1
  Delay22/1 -> [eof]
  Delay24/1 -> [eoh]
  speadizer_vegas_lbw/3(eof) -> Delay22/1
  speadizer_vegas_lbw/4(eoh) -> Delay24/1
  Delay30/1 -> [pkt_data]
  Delay23/1 -> [data_valid_spead]
  [data_valid_spead] -> Delay72/1
  [eof] -> Delay2/1
  Delay31/1 -> Delay18/1
  [cnt_rst] -> Delay31/1
  Delay32/1 -> gbe0/1
  Delay71/1 -> gbe0/2
  Delay72/1 -> gbe0/3
  Delay1/1 -> gbe0/4
  Delay11/1 -> gbe0/5
  Delay2/1 -> gbe0/6
  Delay6/1 -> gbe0/8
  Delay18/1 -> gbe0/7
  Slice13/1 -> [adc_sync]
  Delay91/1 -> [sync_period]
  delay17/1 -> [pol1_re_1]
  delay18/1 -> [pol1_im_1]
  delay19/1 -> [pol2_re_1]
  delay20/1 -> [pol2_im_1]
  gbe0/1 -> Terminator17/1
  gbe0/2 -> Terminator16/1
  gbe0/3 -> Terminator13/1
  gbe0/4 -> Terminator12/1
  gbe0/5 -> [txack]
  gbe0/6 -> Terminator/1
  gbe0/7 -> Terminator11/1
  gbe0/8 -> Terminator10/1
  gbe0/9 -> Terminator4/1
  gbe0/10 -> Terminator1/1
  gbe0/11 -> Terminator9/1
  gbe0/12 -> Terminator8/1
  [SR0] -> bus_create4/3(in3)
  [CAL] -> bus_create4/2(in2)
  [LOC_BLANK] -> bus_create4/1(in1)
  bus_create4/1(bus_out) -> Register24/1
  bus_expand3/2(out3) -> leds_gpios/1(IN0)
  bus_expand3/3(out2) -> leds_gpios/2(IN1)
  bus_expand3/4(lsb_out1) -> leds_gpios/3(IN2)
  err_clear_sim5/1 -> gpio/1
  gpio/1 -> Register22/1, Mux3/4
  [spec_tick] -> Register22/2
  err_clear_sim6/1 -> master_slave_sel/1
  bit 0 and 1/1 -> Mux3/1
  master_slave_sel/1 -> bit 0 and 1/1, bit 2/1
  Mux2/1 -> bus_expand3/1(bus_in)
  bit 2/1 -> [ssg_bits_gpios]
  [ssg_bits_gpios] -> Mux2/1
  Register22/1 -> Mux3/3, Mux2/3
  Register24/1 -> Mux3/2, Mux2/2
  Mux3/1 -> top bit/1, Register25/1
  top bit/1 -> [blank]
  [int_tick] -> Register25/2
  Register25/1 -> [status_bits]
  manrst/1 -> Register8/1
  Constant7/1 -> manrst/1
  Register8/1 -> Slice6/1, Slice9/1
  Slice6/1 -> Delay28/1
  Constant27/1 -> ssg_bram/2(data_in)
  Constant8/1 -> ssg_bram/3(we)
  Counter/1 -> ssg_bram/1(addr)
  ssg_bram/1(data_out) -> SuperSlice/1(In)
  SuperSlice/1(DUR) -> [DUR]
  SuperSlice/2(AS/R) -> [ASR]
  SuperSlice/3(S/R1) -> [SR1]
  SuperSlice/4(S/R0) -> [SR0]
  SuperSlice/5(CAL) -> [CAL]
  SuperSlice/6(BLANK) -> [LOC_BLANK]
  Register10/1 -> Inverter/1
  Constant10/1 -> Register10/1
  sg/1(sync_out) -> [sync_gen]
  sg/2(pps_out) -> [master_rst]
  arm/1 -> Register20/1
  Constant4/1 -> arm/1
  Register20/1 -> Slice8/1
  Slice8/1 -> [arm]
  [arm] -> Register10/2
  [master_rst] -> Register10/3
  [adc_sync] -> sg/1(pps)
  Slice9/1 -> [auto_reset_enable]
  [arm] -> posedge3/1
  posedge3/1 -> Logical2/2
  Logical2/1 -> [tge_rst]
  Logical3/1 -> Logical2/3
  [auto_reset_enable] -> Logical3/1
  [txack] -> posedge1/1
  posedge1/1 -> Logical3/2
  Constant11/1 -> length/1
  length/1 -> cycle_limit/2(cnt_limit)
  dur_len/1(at_limit) -> Counter/2
  cycle_limit/1(at_limit) -> Counter/1
  [DUR] -> dur_len/2(cnt_limit)
  [master_rst] -> dur_len/3(master_rst)
  [master_rst] -> cycle_limit/3(master_rst)
  [spec_tick] -> cycle_limit/1(cnt_en)
  [spec_tick] -> dur_len/1(cnt_en)
  [master_rst] -> Counter1/1
  Counter1/1 -> Slice10/1, Slice11/1
  Slice10/1 -> negedge/1
  Slice11/1 -> [spec_cnt]
  negedge/1 -> [spec_tick]
  [master_rst] -> time_ctr1/1
  Inverter/1 -> [spead_rst]  "ppsready"
  Delay28/1 -> Logical2/1
  [pkt_data] -> Delay25/1
  [data_valid_spead] -> Delay88/1
  [eoh] -> pktsnap/2(trig)
  Delay25/1 -> pktsnap/1(din)
  Delay88/1 -> pktsnap/3(we)
  negedge1/1 -> [int_tick]
  [data_valid] -> Logical/1
  Logical/1 -> negedge1/1
  Constant1/1 -> Logical/2
  Constant9/1 -> bus_create2/1(in1)
  [status_bits] -> bus_create2/2(in2)
  bus_create2/1(bus_out) -> speadizer_vegas_lbw/7(status_bits)

== system v13_na_ri_8r128dr_ver101/SuperSlice ==
  In : Inport
  Slice1 : xbsIndex_r4/Slice  nbits=27  boolean_output=off  mode=Lower Bit Location + Width  bit1=0  base1=MSB of Input  bit0=5  base0=LSB of Input  dbl_ovrd=off  block_type=slice
      settings: infoedit="Extracts a given range of bits from each input sample and presents it at the output.  The output type is ordinarily unsigned with binary point at zero, but can be Boolean when the slice is one bit wide.<P><P>Hardware notes: In hardware this block costs nothing."  block_version=10.1.2
  Slice2 : xbsIndex_r4/Slice  nbits=1  boolean_output=off  mode=Lower Bit Location + Width  bit1=0  base1=MSB of Input  bit0=4  base0=LSB of Input  dbl_ovrd=off  block_type=slice
      settings: infoedit="Extracts a given range of bits from each input sample and presents it at the output.  The output type is ordinarily unsigned with binary point at zero, but can be Boolean when the slice is one bit wide.<P><P>Hardware notes: In hardware this block costs nothing."  block_version=10.1.2
  Slice3 : xbsIndex_r4/Slice  nbits=1  boolean_output=off  mode=Lower Bit Location + Width  bit1=0  base1=MSB of Input  bit0=3  base0=LSB of Input  dbl_ovrd=off  block_type=slice
      settings: infoedit="Extracts a given range of bits from each input sample and presents it at the output.  The output type is ordinarily unsigned with binary point at zero, but can be Boolean when the slice is one bit wide.<P><P>Hardware notes: In hardware this block costs nothing."  block_version=10.1.2
  Slice4 : xbsIndex_r4/Slice  nbits=1  boolean_output=off  mode=Lower Bit Location + Width  bit1=0  base1=MSB of Input  bit0=2  base0=LSB of Input  dbl_ovrd=off  block_type=slice
      settings: infoedit="Extracts a given range of bits from each input sample and presents it at the output.  The output type is ordinarily unsigned with binary point at zero, but can be Boolean when the slice is one bit wide.<P><P>Hardware notes: In hardware this block costs nothing."  block_version=10.1.2
  Slice5 : xbsIndex_r4/Slice  nbits=1  boolean_output=off  mode=Lower Bit Location + Width  bit1=0  base1=MSB of Input  bit0=1  base0=LSB of Input  dbl_ovrd=off  block_type=slice
      settings: block_version=11.3
  Slice6 : xbsIndex_r4/Slice  nbits=1  boolean_output=off  mode=Lower Bit Location + Width  bit1=0  base1=MSB of Input  bit0=0  base0=LSB of Input  dbl_ovrd=off  block_type=slice
      settings: block_version=11.3
  DUR : Outport
  AS/R : Outport  Port=2
  S/R1 : Outport  Port=3
  S/R0 : Outport  Port=4
  CAL : Outport  Port=5
  BLANK : Outport  Port=6
  wires:
  Slice6/1 -> BLANK/1
  Slice5/1 -> CAL/1
  Slice4/1 -> S/R0/1
  Slice3/1 -> S/R1/1
  Slice2/1 -> AS/R/1
  Slice1/1 -> DUR/1
  In/1 -> Slice1/1, Slice2/1, Slice3/1, Slice4/1, Slice5/1, Slice6/1
